simulink model "zcu102_f_200msps_64i_4096c"  (R2021a)
settings: Solver=VariableStepDiscrete  StartTime=0.0  StopTime=10.0  RelTol=1e-3
undefined symbols (not defined in the file or in its folder): datetime, pfb_coeff_gen_calc, posixtime
libraries: xbsIndex_r4 x49646, casper_library_misc x229, casper_library_delays x133, xps_library x20, casper_library_sources x4, xbsReplacements_r4 x4
(part 14 of 502)

== system zcu102_f_200msps_64i_4096c/delay/bus_expand4 ==
  bus_in : Inport
  slice1 : xbsIndex_r4/Slice  nbits=1  boolean_output=on  mode=Upper Bit Location + Width  bit1=-15  base1=MSB of Input  bit0=0  base0=LSB of Input  dbl_ovrd=off  block_type=slice
  slice10 : xbsIndex_r4/Slice  nbits=1  boolean_output=on  mode=Upper Bit Location + Width  bit1=-6  base1=MSB of Input  bit0=0  base0=LSB of Input  dbl_ovrd=off  block_type=slice
  slice11 : xbsIndex_r4/Slice  nbits=1  boolean_output=on  mode=Upper Bit Location + Width  bit1=-5  base1=MSB of Input  bit0=0  base0=LSB of Input  dbl_ovrd=off  block_type=slice
  slice12 : xbsIndex_r4/Slice  nbits=1  boolean_output=on  mode=Upper Bit Location + Width  bit1=-4  base1=MSB of Input  bit0=0  base0=LSB of Input  dbl_ovrd=off  block_type=slice
  slice13 : xbsIndex_r4/Slice  nbits=1  boolean_output=on  mode=Upper Bit Location + Width  bit1=-3  base1=MSB of Input  bit0=0  base0=LSB of Input  dbl_ovrd=off  block_type=slice
  slice14 : xbsIndex_r4/Slice  nbits=1  boolean_output=on  mode=Upper Bit Location + Width  bit1=-2  base1=MSB of Input  bit0=0  base0=LSB of Input  dbl_ovrd=off  block_type=slice
  slice15 : xbsIndex_r4/Slice  nbits=1  boolean_output=on  mode=Upper Bit Location + Width  bit1=-1  base1=MSB of Input  bit0=0  base0=LSB of Input  dbl_ovrd=off  block_type=slice
  slice16 : xbsIndex_r4/Slice  nbits=1  boolean_output=on  mode=Upper Bit Location + Width  bit1=0  base1=MSB of Input  bit0=0  base0=LSB of Input  dbl_ovrd=off  block_type=slice
  slice2 : xbsIndex_r4/Slice  nbits=1  boolean_output=on  mode=Upper Bit Location + Width  bit1=-14  base1=MSB of Input  bit0=0  base0=LSB of Input  dbl_ovrd=off  block_type=slice
  slice3 : xbsIndex_r4/Slice  nbits=1  boolean_output=on  mode=Upper Bit Location + Width  bit1=-13  base1=MSB of Input  bit0=0  base0=LSB of Input  dbl_ovrd=off  block_type=slice
  slice4 : xbsIndex_r4/Slice  nbits=1  boolean_output=on  mode=Upper Bit Location + Width  bit1=-12  base1=MSB of Input  bit0=0  base0=LSB of Input  dbl_ovrd=off  block_type=slice
  slice5 : xbsIndex_r4/Slice  nbits=1  boolean_output=on  mode=Upper Bit Location + Width  bit1=-11  base1=MSB of Input  bit0=0  base0=LSB of Input  dbl_ovrd=off  block_type=slice
  slice6 : xbsIndex_r4/Slice  nbits=1  boolean_output=on  mode=Upper Bit Location + Width  bit1=-10  base1=MSB of Input  bit0=0  base0=LSB of Input  dbl_ovrd=off  block_type=slice
  slice7 : xbsIndex_r4/Slice  nbits=1  boolean_output=on  mode=Upper Bit Location + Width  bit1=-9  base1=MSB of Input  bit0=0  base0=LSB of Input  dbl_ovrd=off  block_type=slice
  slice8 : xbsIndex_r4/Slice  nbits=1  boolean_output=on  mode=Upper Bit Location + Width  bit1=-8  base1=MSB of Input  bit0=0  base0=LSB of Input  dbl_ovrd=off  block_type=slice
  slice9 : xbsIndex_r4/Slice  nbits=1  boolean_output=on  mode=Upper Bit Location + Width  bit1=-7  base1=MSB of Input  bit0=0  base0=LSB of Input  dbl_ovrd=off  block_type=slice
  msb_out16 : Outport
  out15 : Outport  Port=2
  out14 : Outport  Port=3
  out13 : Outport  Port=4
  out12 : Outport  Port=5
  out11 : Outport  Port=6
  out10 : Outport  Port=7
  out9 : Outport  Port=8
  out8 : Outport  Port=9
  out7 : Outport  Port=10
  out6 : Outport  Port=11
  out5 : Outport  Port=12
  out4 : Outport  Port=13
  out3 : Outport  Port=14
  out2 : Outport  Port=15
  lsb_out1 : Outport  Port=16  VectorParamsAs1DForOutWhenUnconnected=off
  wires:
  bus_in/1 -> slice16/1, slice15/1, slice14/1, slice13/1, slice12/1, slice11/1, slice10/1, slice9/1, slice8/1, slice7/1, slice6/1, slice5/1, slice4/1, slice3/1, slice2/1, slice1/1
  slice16/1 -> msb_out16/1
  slice15/1 -> out15/1
  slice14/1 -> out14/1
  slice13/1 -> out13/1
  slice12/1 -> out12/1
  slice11/1 -> out11/1
  slice10/1 -> out10/1
  slice9/1 -> out9/1
  slice8/1 -> out8/1
  slice7/1 -> out7/1
  slice6/1 -> out6/1
  slice5/1 -> out5/1
  slice4/1 -> out4/1
  slice3/1 -> out3/1
  slice2/1 -> out2/1
  slice1/1 -> lsb_out1/1

== system zcu102_f_200msps_64i_4096c/delay/delay0 ==
  sim_1 : Inport
  convert1_1 : DataTypeConversion  OutDataTypeStr=fixdt('ufix13_En0')  LockScale=on
  convert2_1 : DataTypeConversion  OutDataTypeStr=fixdt('ufix13_En0')  ConvertRealWorld=Stored Integer (SI)  LockScale=on
  gain_1 : Gain  OutDataTypeStr=fixdt('ufix32_En0')
  io_delay : xbsIndex_r4/Delay  rst=off  infoeditControl=Selection of Reset will increase slice count due to use of real FFs and instead of SRLs  en=off  latency=0  reg_retiming=on  dbl_ovrd=off  block_type=delay
  reint1 : xbsIndex_r4/Reinterpret  force_arith_type=on  arith_type=Unsigned  force_bin_pt=on  bin_pt=0  block_type=reinterpret
  slice_reg : xbsIndex_r4/Slice  nbits=13  boolean_output=off  mode=Lower Bit Location + Width  bit1=0  base1=MSB of Input  bit0=0  base0=LSB of Input  dbl_ovrd=off  block_type=slice
  zcu102_f_200msps_64i_4096c_delay_delay0_user_data_out : xbsIndex_r4/Gateway In  gui_display_data_type=Fixed-point  arith_type=Unsigned  n_bits=32  bin_pt=0  preci_type=Single  exp_width=8  frac_width=24  rows=1  columns=1  quantization="Round  (unbiased: +/- Inf)"  overflow=Saturate  period=1  UseAsADC=off  ADCChannel='1'  interface=None  automatic_assignment=on  address_offset=hex2dec('00')  intf_name=''  intf_description=''  default_value=0  timing_constraint=None  locs_specified=off  LOCs={}  io_standard={}  inherit_from_input=off  hdl_port=on  dbl_ovrd=off  block_type=gatewayin
  in_reg : Outport  VectorParamsAs1DForOutWhenUnconnected=off
  wires:
  zcu102_f_200msps_64i_4096c_delay_delay0_user_data_out/1 -> io_delay/1
  sim_1/1 -> convert1_1/1
  convert1_1/1 -> convert2_1/1
  convert2_1/1 -> gain_1/1
  gain_1/1 -> zcu102_f_200msps_64i_4096c_delay_delay0_user_data_out/1
  io_delay/1 -> slice_reg/1
  slice_reg/1 -> reint1/1
  reint1/1 -> in_reg/1

== system zcu102_f_200msps_64i_4096c/delay/delay1 ==
  sim_1 : Inport
  convert1_1 : DataTypeConversion  OutDataTypeStr=fixdt('ufix13_En0')  LockScale=on
  convert2_1 : DataTypeConversion  OutDataTypeStr=fixdt('ufix13_En0')  ConvertRealWorld=Stored Integer (SI)  LockScale=on
  gain_1 : Gain  OutDataTypeStr=fixdt('ufix32_En0')
  io_delay : xbsIndex_r4/Delay  rst=off  infoeditControl=Selection of Reset will increase slice count due to use of real FFs and instead of SRLs  en=off  latency=0  reg_retiming=on  dbl_ovrd=off  block_type=delay
  reint1 : xbsIndex_r4/Reinterpret  force_arith_type=on  arith_type=Unsigned  force_bin_pt=on  bin_pt=0  block_type=reinterpret
  slice_reg : xbsIndex_r4/Slice  nbits=13  boolean_output=off  mode=Lower Bit Location + Width  bit1=0  base1=MSB of Input  bit0=0  base0=LSB of Input  dbl_ovrd=off  block_type=slice
  zcu102_f_200msps_64i_4096c_delay_delay1_user_data_out : xbsIndex_r4/Gateway In  gui_display_data_type=Fixed-point  arith_type=Unsigned  n_bits=32  bin_pt=0  preci_type=Single  exp_width=8  frac_width=24  rows=1  columns=1  quantization="Round  (unbiased: +/- Inf)"  overflow=Saturate  period=1  UseAsADC=off  ADCChannel='1'  interface=None  automatic_assignment=on  address_offset=hex2dec('00')  intf_name=''  intf_description=''  default_value=0  timing_constraint=None  locs_specified=off  LOCs={}  io_standard={}  inherit_from_input=off  hdl_port=on  dbl_ovrd=off  block_type=gatewayin
  in_reg : Outport  VectorParamsAs1DForOutWhenUnconnected=off
  wires:
  zcu102_f_200msps_64i_4096c_delay_delay1_user_data_out/1 -> io_delay/1
  sim_1/1 -> convert1_1/1
  convert1_1/1 -> convert2_1/1
  convert2_1/1 -> gain_1/1
  gain_1/1 -> zcu102_f_200msps_64i_4096c_delay_delay1_user_data_out/1
  io_delay/1 -> slice_reg/1
  slice_reg/1 -> reint1/1
  reint1/1 -> in_reg/1

== system zcu102_f_200msps_64i_4096c/delay/delay_en0 ==
  sim_1 : Inport
  convert1_1 : DataTypeConversion  OutDataTypeStr=fixdt('ufix32_En0')  LockScale=on
  convert2_1 : DataTypeConversion  OutDataTypeStr=fixdt('ufix32_En0')  ConvertRealWorld=Stored Integer (SI)  LockScale=on
  gain_1 : Gain  OutDataTypeStr=fixdt('ufix32_En0')
  io_delay : xbsIndex_r4/Delay  rst=off  infoeditControl=Selection of Reset will increase slice count due to use of real FFs and instead of SRLs  en=off  latency=0  reg_retiming=on  dbl_ovrd=off  block_type=delay
  reint1 : xbsIndex_r4/Reinterpret  force_arith_type=on  arith_type=Unsigned  force_bin_pt=on  bin_pt=0  block_type=reinterpret
  slice_reg : xbsIndex_r4/Slice  nbits=32  boolean_output=off  mode=Lower Bit Location + Width  bit1=0  base1=MSB of Input  bit0=0  base0=LSB of Input  dbl_ovrd=off  block_type=slice
  zcu102_f_200msps_64i_4096c_delay_delay_en0_user_data_out : xbsIndex_r4/Gateway In  gui_display_data_type=Fixed-point  arith_type=Unsigned  n_bits=32  bin_pt=0  preci_type=Single  exp_width=8  frac_width=24  rows=1  columns=1  quantization="Round  (unbiased: +/- Inf)"  overflow=Saturate  period=1  UseAsADC=off  ADCChannel='1'  interface=None  automatic_assignment=on  address_offset=hex2dec('00')  intf_name=''  intf_description=''  default_value=0  timing_constraint=None  locs_specified=off  LOCs={}  io_standard={}  inherit_from_input=off  hdl_port=on  dbl_ovrd=off  block_type=gatewayin
  in_reg : Outport  VectorParamsAs1DForOutWhenUnconnected=off
  wires:
  zcu102_f_200msps_64i_4096c_delay_delay_en0_user_data_out/1 -> io_delay/1
  sim_1/1 -> convert1_1/1
  convert1_1/1 -> convert2_1/1
  convert2_1/1 -> gain_1/1
  gain_1/1 -> zcu102_f_200msps_64i_4096c_delay_delay_en0_user_data_out/1
  io_delay/1 -> slice_reg/1
  slice_reg/1 -> reint1/1
  reint1/1 -> in_reg/1

== system zcu102_f_200msps_64i_4096c/delay/delay_en1 ==
  sim_1 : Inport
  convert1_1 : DataTypeConversion  OutDataTypeStr=fixdt('ufix32_En0')  LockScale=on
  convert2_1 : DataTypeConversion  OutDataTypeStr=fixdt('ufix32_En0')  ConvertRealWorld=Stored Integer (SI)  LockScale=on
  gain_1 : Gain  OutDataTypeStr=fixdt('ufix32_En0')
  io_delay : xbsIndex_r4/Delay  rst=off  infoeditControl=Selection of Reset will increase slice count due to use of real FFs and instead of SRLs  en=off  latency=0  reg_retiming=on  dbl_ovrd=off  block_type=delay
  reint1 : xbsIndex_r4/Reinterpret  force_arith_type=on  arith_type=Unsigned  force_bin_pt=on  bin_pt=0  block_type=reinterpret
  slice_reg : xbsIndex_r4/Slice  nbits=32  boolean_output=off  mode=Lower Bit Location + Width  bit1=0  base1=MSB of Input  bit0=0  base0=LSB of Input  dbl_ovrd=off  block_type=slice
  zcu102_f_200msps_64i_4096c_delay_delay_en1_user_data_out : xbsIndex_r4/Gateway In  gui_display_data_type=Fixed-point  arith_type=Unsigned  n_bits=32  bin_pt=0  preci_type=Single  exp_width=8  frac_width=24  rows=1  columns=1  quantization="Round  (unbiased: +/- Inf)"  overflow=Saturate  period=1  UseAsADC=off  ADCChannel='1'  interface=None  automatic_assignment=on  address_offset=hex2dec('00')  intf_name=''  intf_description=''  default_value=0  timing_constraint=None  locs_specified=off  LOCs={}  io_standard={}  inherit_from_input=off  hdl_port=on  dbl_ovrd=off  block_type=gatewayin
  in_reg : Outport  VectorParamsAs1DForOutWhenUnconnected=off
  wires:
  zcu102_f_200msps_64i_4096c_delay_delay_en1_user_data_out/1 -> io_delay/1
  sim_1/1 -> convert1_1/1
  convert1_1/1 -> convert2_1/1
  convert2_1/1 -> gain_1/1
  gain_1/1 -> zcu102_f_200msps_64i_4096c_delay_delay_en1_user_data_out/1
  io_delay/1 -> slice_reg/1
  slice_reg/1 -> reint1/1
  reint1/1 -> in_reg/1

== system zcu102_f_200msps_64i_4096c/delay/delay_store0 ==
  delay_in : Inport
  en0 : Inport  Port=2
  en1 : Inport  Port=3
  en2 : Inport  Port=4
  en3 : Inport  Port=5
  en4 : Inport  Port=6
  en5 : Inport  Port=7
  en6 : Inport  Port=8
  en7 : Inport  Port=9
  en8 : Inport  Port=10
  en9 : Inport  Port=11
  en10 : Inport  Port=12
  en11 : Inport  Port=13
  en12 : Inport  Port=14
  en13 : Inport  Port=15
  en14 : Inport  Port=16
  en15 : Inport  Port=17
  en16 : Inport  Port=18
  en17 : Inport  Port=19
  en18 : Inport  Port=20
  en19 : Inport  Port=21
  en20 : Inport  Port=22
  en21 : Inport  Port=23
  en22 : Inport  Port=24
  en23 : Inport  Port=25
  en24 : Inport  Port=26
  en25 : Inport  Port=27
  en26 : Inport  Port=28
  en27 : Inport  Port=29
  en28 : Inport  Port=30
  en29 : Inport  Port=31
  en30 : Inport  Port=32
  en31 : Inport  Port=33
  Mux{,1..3} (4 alike) : xbsIndex_r4/Mux  inputs=8  en=off  latency=1  precision=Full  arith_type=Unsigned  n_bits=16  bin_pt=14  quantization=Truncate  overflow=Wrap  dbl_ovrd=off  block_type=mux
  Mux4 : xbsIndex_r4/Mux  inputs=4  en=off  latency=1  precision=Full  arith_type=Unsigned  n_bits=16  bin_pt=14  quantization=Truncate  overflow=Wrap  dbl_ovrd=off  block_type=mux
  Register{,1..31} (32 alike) : xbsIndex_r4/Register  init=0  rst=off  en=on  dbl_ovrd=off  block_type=register
  Slice : xbsIndex_r4/Slice  nbits=3  boolean_output=off  mode=Lower Bit Location + Width  bit1=0  base1=MSB of Input  bit0=0  base0=LSB of Input  dbl_ovrd=off  block_type=slice
  Slice1 : xbsIndex_r4/Slice  nbits=2  boolean_output=off  mode=Lower Bit Location + Width  bit1=3  base1=MSB of Input  bit0=3  base0=LSB of Input  dbl_ovrd=off  block_type=slice
  readout : Subsystem  Tag=xps:sw_reg  AncestorBlock=xps_library/Memory/software_register  mask(swreg){io_dir=To Processor, io_delay=0, init_val=0, sample_period=1, names=reg, bitwidths=13, bin_pts=0, arith_types=0, sim_port=on, show_format=on, desc_str=""}
      mask prompts: io_dir "I/O direction"; io_delay "I/O delay"; init_val "Initial Value"; sample_period "Sample period"; names "Bitfield names [msb...lsb]"; bitwidths "Bitfield widths"; bin_pts "Bitfield binary pts"; arith_types "Bitfield types, ufix=0, fix=1, bool=2"; sim_port "Provide sim input/output?"; show_format "Print format string?"; desc_str "Description"
      mask help: A 32-bit software-accessible register. Can be divided into bitfields of varying widths and types using the fields in the block mask.
      mask init:
      | swreg_init(gcb)
      settings: AttributesFormatString=1output:uf13.0 = 13 bits
      mask TypeOptions io_dir: To Processor | From Processor
      mask Callback io_dir:
      | mask_enables = get_param(gcb, 'MaskEnables');
      | mask_names = get_param(gcb, 'MaskNames');
      | if strcmp(get_param(gcb, 'io_dir'), 'To Processor'),
      |     mask_enables{ismember(mask_names, 'sample_period')} = 'off';
      | else
      |     mask_enables{ismember(mask_names, 'sample_period')} = 'on';
      | end
      | set_param(gcb, 'MaskEnables', mask_enables);
      mask DialogControl ParameterTabVar0 Prompt: Setup
  sel : Subsystem  Tag=xps:sw_reg  AncestorBlock=xps_library/Memory/software_register  mask(swreg){io_dir=From Processor, io_delay=0, init_val=0, sample_period=1, names=reg, bitwidths=5, bin_pts=0, arith_types=0, sim_port=on, show_format=on, desc_str=""}
      mask prompts: io_dir "I/O direction"; io_delay "I/O delay"; init_val "Initial Value"; sample_period "Sample period"; names "Bitfield names [msb...lsb]"; bitwidths "Bitfield widths"; bin_pts "Bitfield binary pts"; arith_types "Bitfield types, ufix=0, fix=1, bool=2"; sim_port "Provide sim input/output?"; show_format "Print format string?"; desc_str "Description"
      mask help: A 32-bit software-accessible register. Can be divided into bitfields of varying widths and types using the fields in the block mask.
      mask init:
      | swreg_init(gcb)
      settings: AttributesFormatString=1input:uf5.0 = 5 bits
      mask TypeOptions io_dir: To Processor | From Processor
      mask Callback io_dir:
      | mask_enables = get_param(gcb, 'MaskEnables');
      | mask_names = get_param(gcb, 'MaskNames');
      | if strcmp(get_param(gcb, 'io_dir'), 'To Processor'),
      |     mask_enables{ismember(mask_names, 'sample_period')} = 'off';
      | else
      |     mask_enables{ismember(mask_names, 'sample_period')} = 'on';
      | end
      | set_param(gcb, 'MaskEnables', mask_enables);
      mask DialogControl ParameterTabVar0 Prompt: Setup
  delay0 : Outport  VectorParamsAs1DForOutWhenUnconnected=off
  delay1 : Outport  Port=2  VectorParamsAs1DForOutWhenUnconnected=off
  delay2 : Outport  Port=3  VectorParamsAs1DForOutWhenUnconnected=off
  delay3 : Outport  Port=4  VectorParamsAs1DForOutWhenUnconnected=off
  delay4 : Outport  Port=5  VectorParamsAs1DForOutWhenUnconnected=off
  delay5 : Outport  Port=6  VectorParamsAs1DForOutWhenUnconnected=off
  delay6 : Outport  Port=7  VectorParamsAs1DForOutWhenUnconnected=off
  delay7 : Outport  Port=8  VectorParamsAs1DForOutWhenUnconnected=off
  delay8 : Outport  Port=9  VectorParamsAs1DForOutWhenUnconnected=off
  delay9 : Outport  Port=10  VectorParamsAs1DForOutWhenUnconnected=off
  delay10 : Outport  Port=11  VectorParamsAs1DForOutWhenUnconnected=off
  delay11 : Outport  Port=12  VectorParamsAs1DForOutWhenUnconnected=off
  delay12 : Outport  Port=13  VectorParamsAs1DForOutWhenUnconnected=off
  delay13 : Outport  Port=14  VectorParamsAs1DForOutWhenUnconnected=off
  delay14 : Outport  Port=15  VectorParamsAs1DForOutWhenUnconnected=off
  delay15 : Outport  Port=16  VectorParamsAs1DForOutWhenUnconnected=off
  delay16 : Outport  Port=17  VectorParamsAs1DForOutWhenUnconnected=off
  delay17 : Outport  Port=18  VectorParamsAs1DForOutWhenUnconnected=off
  delay18 : Outport  Port=19  VectorParamsAs1DForOutWhenUnconnected=off
  delay19 : Outport  Port=20  VectorParamsAs1DForOutWhenUnconnected=off
  delay20 : Outport  Port=21  VectorParamsAs1DForOutWhenUnconnected=off
  delay21 : Outport  Port=22  VectorParamsAs1DForOutWhenUnconnected=off
  delay22 : Outport  Port=23  VectorParamsAs1DForOutWhenUnconnected=off
  delay23 : Outport  Port=24  VectorParamsAs1DForOutWhenUnconnected=off
  delay24 : Outport  Port=25  VectorParamsAs1DForOutWhenUnconnected=off
  delay25 : Outport  Port=26  VectorParamsAs1DForOutWhenUnconnected=off
  delay26 : Outport  Port=27  VectorParamsAs1DForOutWhenUnconnected=off
  delay27 : Outport  Port=28  VectorParamsAs1DForOutWhenUnconnected=off
  delay28 : Outport  Port=29  VectorParamsAs1DForOutWhenUnconnected=off
  delay29 : Outport  Port=30  VectorParamsAs1DForOutWhenUnconnected=off
  delay30 : Outport  Port=31  VectorParamsAs1DForOutWhenUnconnected=off
  delay31 : Outport  Port=32  VectorParamsAs1DForOutWhenUnconnected=off
  wires:
  Register/1 -> Mux/2, delay0/1
  en0/1 -> Register/2
  delay_in/1 -> [delay]
  [delay] -> Register/1
  [delay] -> Register1/1
  en1/1 -> Register1/2
  Register1/1 -> Mux/3, delay1/1
  Register2/1 -> Mux/4, delay2/1
  en2/1 -> Register2/2
  [delay] -> Register2/1
  [delay] -> Register3/1
  en3/1 -> Register3/2
  Register3/1 -> Mux/5, delay3/1
  Register4/1 -> Mux/6, delay4/1
  en4/1 -> Register4/2
  [delay] -> Register4/1
  [delay] -> Register5/1
  en5/1 -> Register5/2
  Register5/1 -> Mux/7, delay5/1
  Register6/1 -> Mux/8, delay6/1
  en6/1 -> Register6/2
  [delay] -> Register6/1
  [delay] -> Register7/1
  en7/1 -> Register7/2
  Register7/1 -> Mux/9, delay7/1
  en15/1 -> Register15/2
  [delay] -> Register15/1
  [delay] -> Register14/1
  en14/1 -> Register14/2
  en13/1 -> Register13/2
  [delay] -> Register13/1
  [delay] -> Register12/1
  en12/1 -> Register12/2
  en11/1 -> Register11/2
  [delay] -> Register11/1
  [delay] -> Register10/1
  en10/1 -> Register10/2
  en9/1 -> Register9/2
  [delay] -> Register9/1
  [delay] -> Register8/1
  en8/1 -> Register8/2
  en16/1 -> Register22/2
  [delay] -> Register22/1
  [delay] -> Register23/1
  en17/1 -> Register23/2
  en18/1 -> Register16/2
  [delay] -> Register16/1
  [delay] -> Register17/1
  en19/1 -> Register17/2
  en20/1 -> Register18/2
  [delay] -> Register18/1
  [delay] -> Register19/1
  en21/1 -> Register19/2
  en22/1 -> Register20/2
  [delay] -> Register20/1
  [delay] -> Register21/1
  en23/1 -> Register21/2
  en31/1 -> Register29/2
  [delay] -> Register29/1
  [delay] -> Register28/1
  en30/1 -> Register28/2
  en29/1 -> Register27/2
  [delay] -> Register27/1
  [delay] -> Register26/1
  en28/1 -> Register26/2
  en27/1 -> Register25/2
  [delay] -> Register25/1
  [delay] -> Register24/1
  en26/1 -> Register24/2
  en25/1 -> Register31/2
  [delay] -> Register31/1
  [delay] -> Register30/1
  en24/1 -> Register30/2
  Register15/1 -> Mux1/9, delay15/1
  Register14/1 -> Mux1/8, delay14/1
  Register13/1 -> Mux1/7, delay13/1
  Register12/1 -> Mux1/6, delay12/1
  Register11/1 -> Mux1/5, delay11/1
  Register10/1 -> Mux1/4, delay10/1
  Register9/1 -> Mux1/3, delay9/1
  Register8/1 -> Mux1/2, delay8/1
  Register22/1 -> Mux2/2, delay16/1
  Register23/1 -> delay17/1, Mux2/3
  Register16/1 -> delay18/1, Mux2/4
  Register17/1 -> Mux2/5, delay19/1
  Register18/1 -> Mux2/6, delay20/1
  Register19/1 -> Mux2/7, delay21/1
  Register20/1 -> Mux2/8, delay22/1
  Register21/1 -> Mux2/9, delay23/1
  Register29/1 -> Mux3/9, delay31/1
  Register28/1 -> Mux3/8, delay30/1
  Register27/1 -> Mux3/7, delay29/1
  Register26/1 -> Mux3/6, delay28/1
  Register25/1 -> Mux3/5, delay27/1
  Register24/1 -> Mux3/4, delay26/1
  Register31/1 -> Mux3/3, delay25/1
  Register30/1 -> Mux3/2, delay24/1
  Mux/1 -> Mux4/2
  Mux1/1 -> Mux4/3
  Mux2/1 -> Mux4/4
  Mux3/1 -> Mux4/5
  Mux4/1 -> readout/1(out_reg)
  sel/1(in_reg) -> Slice1/1, Slice/1
  Slice1/1 -> [sel_msb]
  Slice/1 -> [sel_lsb]
  [sel_lsb] -> Mux/1
  [sel_lsb] -> Mux1/1
  [sel_lsb] -> Mux2/1
  [sel_lsb] -> Mux3/1
  [sel_msb] -> Mux4/1

== system zcu102_f_200msps_64i_4096c/delay/delay_store1 ==
  delay_in : Inport
  en0 : Inport  Port=2
  en1 : Inport  Port=3
  en2 : Inport  Port=4
  en3 : Inport  Port=5
  en4 : Inport  Port=6
  en5 : Inport  Port=7
  en6 : Inport  Port=8
  en7 : Inport  Port=9
  en8 : Inport  Port=10
  en9 : Inport  Port=11
  en10 : Inport  Port=12
  en11 : Inport  Port=13
  en12 : Inport  Port=14
  en13 : Inport  Port=15
  en14 : Inport  Port=16
  en15 : Inport  Port=17
  en16 : Inport  Port=18
  en17 : Inport  Port=19
  en18 : Inport  Port=20
  en19 : Inport  Port=21
  en20 : Inport  Port=22
  en21 : Inport  Port=23
  en22 : Inport  Port=24
  en23 : Inport  Port=25
  en24 : Inport  Port=26
  en25 : Inport  Port=27
  en26 : Inport  Port=28
  en27 : Inport  Port=29
  en28 : Inport  Port=30
  en29 : Inport  Port=31
  en30 : Inport  Port=32
  en31 : Inport  Port=33
  Mux{,1..3} (4 alike) : xbsIndex_r4/Mux  inputs=8  en=off  latency=1  precision=Full  arith_type=Unsigned  n_bits=16  bin_pt=14  quantization=Truncate  overflow=Wrap  dbl_ovrd=off  block_type=mux
  Mux4 : xbsIndex_r4/Mux  inputs=4  en=off  latency=1  precision=Full  arith_type=Unsigned  n_bits=16  bin_pt=14  quantization=Truncate  overflow=Wrap  dbl_ovrd=off  block_type=mux
  Register{,1..31} (32 alike) : xbsIndex_r4/Register  init=0  rst=off  en=on  dbl_ovrd=off  block_type=register
  Slice : xbsIndex_r4/Slice  nbits=3  boolean_output=off  mode=Lower Bit Location + Width  bit1=0  base1=MSB of Input  bit0=0  base0=LSB of Input  dbl_ovrd=off  block_type=slice
  Slice1 : xbsIndex_r4/Slice  nbits=2  boolean_output=off  mode=Lower Bit Location + Width  bit1=3  base1=MSB of Input  bit0=3  base0=LSB of Input  dbl_ovrd=off  block_type=slice
  readout : Subsystem  Tag=xps:sw_reg  AncestorBlock=xps_library/Memory/software_register  mask(swreg){io_dir=To Processor, io_delay=0, init_val=0, sample_period=1, names=reg, bitwidths=13, bin_pts=0, arith_types=0, sim_port=on, show_format=on, desc_str=""}
      mask prompts: io_dir "I/O direction"; io_delay "I/O delay"; init_val "Initial Value"; sample_period "Sample period"; names "Bitfield names [msb...lsb]"; bitwidths "Bitfield widths"; bin_pts "Bitfield binary pts"; arith_types "Bitfield types, ufix=0, fix=1, bool=2"; sim_port "Provide sim input/output?"; show_format "Print format string?"; desc_str "Description"
      mask help: A 32-bit software-accessible register. Can be divided into bitfields of varying widths and types using the fields in the block mask.
      mask init:
      | swreg_init(gcb)
      settings: AttributesFormatString=1output:uf13.0 = 13 bits
      mask TypeOptions io_dir: To Processor | From Processor
      mask Callback io_dir:
      | mask_enables = get_param(gcb, 'MaskEnables');
      | mask_names = get_param(gcb, 'MaskNames');
      | if strcmp(get_param(gcb, 'io_dir'), 'To Processor'),
      |     mask_enables{ismember(mask_names, 'sample_period')} = 'off';
      | else
      |     mask_enables{ismember(mask_names, 'sample_period')} = 'on';
      | end
      | set_param(gcb, 'MaskEnables', mask_enables);
      mask DialogControl ParameterTabVar0 Prompt: Setup
  sel : Subsystem  Tag=xps:sw_reg  AncestorBlock=xps_library/Memory/software_register  mask(swreg){io_dir=From Processor, io_delay=0, init_val=0, sample_period=1, names=reg, bitwidths=5, bin_pts=0, arith_types=0, sim_port=on, show_format=on, desc_str=""}
      mask prompts: io_dir "I/O direction"; io_delay "I/O delay"; init_val "Initial Value"; sample_period "Sample period"; names "Bitfield names [msb...lsb]"; bitwidths "Bitfield widths"; bin_pts "Bitfield binary pts"; arith_types "Bitfield types, ufix=0, fix=1, bool=2"; sim_port "Provide sim input/output?"; show_format "Print format string?"; desc_str "Description"
      mask help: A 32-bit software-accessible register. Can be divided into bitfields of varying widths and types using the fields in the block mask.
      mask init:
      | swreg_init(gcb)
      settings: AttributesFormatString=1input:uf5.0 = 5 bits
      mask TypeOptions io_dir: To Processor | From Processor
      mask Callback io_dir:
      | mask_enables = get_param(gcb, 'MaskEnables');
      | mask_names = get_param(gcb, 'MaskNames');
      | if strcmp(get_param(gcb, 'io_dir'), 'To Processor'),
      |     mask_enables{ismember(mask_names, 'sample_period')} = 'off';
      | else
      |     mask_enables{ismember(mask_names, 'sample_period')} = 'on';
      | end
      | set_param(gcb, 'MaskEnables', mask_enables);
      mask DialogControl ParameterTabVar0 Prompt: Setup
  delay0 : Outport  VectorParamsAs1DForOutWhenUnconnected=off
  delay1 : Outport  Port=2  VectorParamsAs1DForOutWhenUnconnected=off
  delay2 : Outport  Port=3  VectorParamsAs1DForOutWhenUnconnected=off
  delay3 : Outport  Port=4  VectorParamsAs1DForOutWhenUnconnected=off
  delay4 : Outport  Port=5  VectorParamsAs1DForOutWhenUnconnected=off
  delay5 : Outport  Port=6  VectorParamsAs1DForOutWhenUnconnected=off
  delay6 : Outport  Port=7  VectorParamsAs1DForOutWhenUnconnected=off
  delay7 : Outport  Port=8  VectorParamsAs1DForOutWhenUnconnected=off
  delay8 : Outport  Port=9  VectorParamsAs1DForOutWhenUnconnected=off
  delay9 : Outport  Port=10  VectorParamsAs1DForOutWhenUnconnected=off
  delay10 : Outport  Port=11  VectorParamsAs1DForOutWhenUnconnected=off
  delay11 : Outport  Port=12  VectorParamsAs1DForOutWhenUnconnected=off
  delay12 : Outport  Port=13  VectorParamsAs1DForOutWhenUnconnected=off
  delay13 : Outport  Port=14  VectorParamsAs1DForOutWhenUnconnected=off
  delay14 : Outport  Port=15  VectorParamsAs1DForOutWhenUnconnected=off
  delay15 : Outport  Port=16  VectorParamsAs1DForOutWhenUnconnected=off
  delay16 : Outport  Port=17  VectorParamsAs1DForOutWhenUnconnected=off
  delay17 : Outport  Port=18  VectorParamsAs1DForOutWhenUnconnected=off
  delay18 : Outport  Port=19  VectorParamsAs1DForOutWhenUnconnected=off
  delay19 : Outport  Port=20  VectorParamsAs1DForOutWhenUnconnected=off
  delay20 : Outport  Port=21  VectorParamsAs1DForOutWhenUnconnected=off
  delay21 : Outport  Port=22  VectorParamsAs1DForOutWhenUnconnected=off
  delay22 : Outport  Port=23  VectorParamsAs1DForOutWhenUnconnected=off
  delay23 : Outport  Port=24  VectorParamsAs1DForOutWhenUnconnected=off
  delay24 : Outport  Port=25  VectorParamsAs1DForOutWhenUnconnected=off
  delay25 : Outport  Port=26  VectorParamsAs1DForOutWhenUnconnected=off
  delay26 : Outport  Port=27  VectorParamsAs1DForOutWhenUnconnected=off
  delay27 : Outport  Port=28  VectorParamsAs1DForOutWhenUnconnected=off
  delay28 : Outport  Port=29  VectorParamsAs1DForOutWhenUnconnected=off
  delay29 : Outport  Port=30  VectorParamsAs1DForOutWhenUnconnected=off
  delay30 : Outport  Port=31  VectorParamsAs1DForOutWhenUnconnected=off
  delay31 : Outport  Port=32  VectorParamsAs1DForOutWhenUnconnected=off
  wires:
  [sel_msb] -> Mux4/1
  [sel_lsb] -> Mux3/1
  [sel_lsb] -> Mux2/1
  [sel_lsb] -> Mux1/1
  [sel_lsb] -> Mux/1
  Slice/1 -> [sel_lsb]
  Slice1/1 -> [sel_msb]
  sel/1(in_reg) -> Slice/1, Slice1/1
  Mux4/1 -> readout/1(out_reg)
  Mux3/1 -> Mux4/5
  Mux2/1 -> Mux4/4
  Mux1/1 -> Mux4/3
  Mux/1 -> Mux4/2
  Register30/1 -> delay24/1, Mux3/2
  Register31/1 -> delay25/1, Mux3/3
  Register24/1 -> delay26/1, Mux3/4
  Register25/1 -> delay27/1, Mux3/5
  Register26/1 -> delay28/1, Mux3/6
  Register27/1 -> delay29/1, Mux3/7
  Register28/1 -> delay30/1, Mux3/8
  Register29/1 -> delay31/1, Mux3/9
  Register21/1 -> delay23/1, Mux2/9
  Register20/1 -> delay22/1, Mux2/8
  Register19/1 -> delay21/1, Mux2/7
  Register18/1 -> delay20/1, Mux2/6
  Register17/1 -> delay19/1, Mux2/5
  Register16/1 -> Mux2/4, delay18/1
  Register23/1 -> Mux2/3, delay17/1
  Register22/1 -> delay16/1, Mux2/2
  Register8/1 -> delay8/1, Mux1/2
  Register9/1 -> delay9/1, Mux1/3
  Register10/1 -> delay10/1, Mux1/4
  Register11/1 -> delay11/1, Mux1/5
  Register12/1 -> delay12/1, Mux1/6
  Register13/1 -> delay13/1, Mux1/7
  Register14/1 -> delay14/1, Mux1/8
  Register15/1 -> delay15/1, Mux1/9
  en24/1 -> Register30/2
  [delay] -> Register30/1
  [delay] -> Register31/1
  en25/1 -> Register31/2
  en26/1 -> Register24/2
  [delay] -> Register24/1
  [delay] -> Register25/1
  en27/1 -> Register25/2
  en28/1 -> Register26/2
  [delay] -> Register26/1
  [delay] -> Register27/1
  en29/1 -> Register27/2
  en30/1 -> Register28/2
  [delay] -> Register28/1
  [delay] -> Register29/1
  en31/1 -> Register29/2
  en23/1 -> Register21/2
  [delay] -> Register21/1
  [delay] -> Register20/1
  en22/1 -> Register20/2
  en21/1 -> Register19/2
  [delay] -> Register19/1
  [delay] -> Register18/1
  en20/1 -> Register18/2
  en19/1 -> Register17/2
  [delay] -> Register17/1
  [delay] -> Register16/1
  en18/1 -> Register16/2
  en17/1 -> Register23/2
  [delay] -> Register23/1
  [delay] -> Register22/1
  en16/1 -> Register22/2
  en8/1 -> Register8/2
  [delay] -> Register8/1
  [delay] -> Register9/1
  en9/1 -> Register9/2
  en10/1 -> Register10/2
  [delay] -> Register10/1
  [delay] -> Register11/1
  en11/1 -> Register11/2
  en12/1 -> Register12/2
  [delay] -> Register12/1
  [delay] -> Register13/1
  en13/1 -> Register13/2
  en14/1 -> Register14/2
  [delay] -> Register14/1
  [delay] -> Register15/1
  en15/1 -> Register15/2
  Register7/1 -> delay7/1, Mux/9
  en7/1 -> Register7/2
  [delay] -> Register7/1
  [delay] -> Register6/1
  en6/1 -> Register6/2
  Register6/1 -> delay6/1, Mux/8
  Register5/1 -> delay5/1, Mux/7
  en5/1 -> Register5/2
  [delay] -> Register5/1
  [delay] -> Register4/1
  en4/1 -> Register4/2
  Register4/1 -> delay4/1, Mux/6
  Register3/1 -> delay3/1, Mux/5
  en3/1 -> Register3/2
  [delay] -> Register3/1
  [delay] -> Register2/1
  en2/1 -> Register2/2
  Register2/1 -> delay2/1, Mux/4
  Register1/1 -> delay1/1, Mux/3
  en1/1 -> Register1/2
  [delay] -> Register1/1
  [delay] -> Register/1
  delay_in/1 -> [delay]
  en0/1 -> Register/2
  Register/1 -> delay0/1, Mux/2

== system zcu102_f_200msps_64i_4096c/delay/delays ==
  addr : Inport
  data_in : Inport  Port=2
  we : Inport  Port=3
  Terminator : Terminator
  Terminator1 : Terminator
  Terminator2 : Terminator
  calc_add : Subsystem  mask{data_width=32, addr_width=6, sample_rate=sample_rate}
      mask prompts: data_width "data width"; addr_width "address width"; sample_rate "sample rate"
  convert_addr : xbsIndex_r4/Convert  gui_display_data_type=Fixed-point  arith_type=Unsigned  n_bits=addr_width  bin_pt=0  float_type=Single  exp_bits=8  fraction_bits=24  quantization=Truncate  overflow=Wrap  en=off  latency=0  pipeline=off  dbl_ovrd=off  block_type=convert
      settings: xl_area=[0, 0, 0, 0, 0, 0, 0]
  convert_din1 : xbsIndex_r4/Convert  gui_display_data_type=Fixed-point  arith_type=Unsigned  n_bits=32  bin_pt=0  float_type=Single  exp_bits=8  fraction_bits=24  quantization=Truncate  overflow=Wrap  en=off  latency=0  pipeline=off  dbl_ovrd=off  block_type=convert
      settings: xl_area=[0, 0, 0, 0, 0, 0, 0]
  convert_we : xbsIndex_r4/Convert  gui_display_data_type=Fixed-point  arith_type=Unsigned  n_bits=1  bin_pt=0  float_type=Single  exp_bits=8  fraction_bits=24  quantization=Truncate  overflow=Wrap  en=off  latency=0  pipeline=off  dbl_ovrd=off  block_type=convert
      settings: xl_area=[0, 0, 0, 0, 0, 0, 0]
  munge_in : Subsystem  AncestorBlock=casper_library_flow_control/munge  mask(munge){divisions=1, div_size=32, order=[0], arith_type_out=Unsigned, bin_pt_out=0}
      mask prompts: divisions "Number of divisions"; div_size "Division size (bits)"; order "Division packing order"; arith_type_out "Output arithmetic type"; bin_pt_out "Output binary point"
      mask help: Splits an input bus into the specified number of divisions and then recombines them in the order specified (most significant division = 0,least = number divisions-1)
      mask init:
      | munge_init(gcb, ...
      |     'divisions', divisions, ...
      |     'div_size', div_size, ...
      |     'order', order, ...
      |     'arith_type_out', arith_type_out, ...
      |     'bin_pt_out', bin_pt_out);
      settings: AttributesFormatString=|
      | split:32
      | join:0
      | Unsigned [32,0]
      mask TypeOptions arith_type_out: Unsigned | Signed  (2's comp) | Floating-point
  munge_out : Subsystem  AncestorBlock=casper_library_flow_control/munge  mask(munge){divisions=1, div_size=32, order=[0], arith_type_out=Unsigned, bin_pt_out=0}
      mask prompts: divisions "Number of divisions"; div_size "Division size (bits)"; order "Division packing order"; arith_type_out "Output arithmetic type"; bin_pt_out "Output binary point"
      mask help: Splits an input bus into the specified number of divisions and then recombines them in the order specified (most significant division = 0,least = number divisions-1)
      mask init:
      | munge_init(gcb, ...
      |     'divisions', divisions, ...
      |     'div_size', div_size, ...
      |     'order', order, ...
      |     'arith_type_out', arith_type_out, ...
      |     'bin_pt_out', bin_pt_out);
      settings: AttributesFormatString=|
      | split:32
      | join:0
      | Unsigned [32,0]
      mask TypeOptions arith_type_out: Unsigned | Signed  (2's comp) | Floating-point
  sim_mem : Subsystem  mask{arith_type=Unsigned, addr_width=6, data_width=32, data_bin_pt=0, init_vals=[0   1   2   3   4   5   6   7   8   9  10  11  12  13  14  15  16  17  18  19  20  21  22  23  24  25  26  27  28  29  30  31  32  33  34  35  36  37  38  39  40  41  42  43  44  45  46  47  48  49  50  51  52  53  54  55  56  57  58  59  60  61  62  63], sample_rate=1, csp_latency=1}
      mask prompts: arith_type "Output Data Type"; addr_width "Address width"; data_width "Data width"; data_bin_pt "Data Binary Point"; init_vals "Initial values"; sample_rate "Sample rate"; csp_latency "Latency"
      mask init:
      | % set_param([gcb, '/data_to_xilinx'], 'arith_type', arith_type)
      | % set_param([gcb, '/data_to_xilinx'], 'n_bits', get_param(gcb, 'data_width'))
      mask TypeOptions arith_type: Unsigned | Signed  (2's comp)
      mask TypeOptions data_width: 8 | 16 | 32 | 64 | 128
  snap2_f_200msps_64i_4096c_delay_delays_addr : xbsIndex_r4/Gateway Out  inherit_from_input=off  hdl_port=on  rows=1  columns=1  UseAsDAC=off  DACChannel='1'  interface=None  automatic_assignment=on  address_offset=hex2dec('00')  intf_name=''  intf_description=''  timing_constraint=None  locs_specified=off  LOCs={'A5','B9','A9','C1','A3','G6','E9','A6','A7','A8','B8','B1','B3','D9','B5','B4','B6','C2'}  io_standard={}  block_type=gatewayout
      settings: infoedit="Gateway out block.  Converts Xilinx fixed point inputs into ouputs of type Simulink integer, double, or fixed point.<P><P>Hardware notes:  In hardware these blocks become top level output ports or are discarded, depending on how they are configured."  sginterface={'Nallatech'=>{'xdspkit'=>{'non_memory_mapped_port'=>'uprev_Shared_BRAM_addr'}},'Xilinx'=>{'jtaghwcosim'=>{'non_memory_mapped_port'=>'uprev_Shared_BRAM_addr'},'xdspkit'=>{'non_memory_mapped_port'=>'uprev_Shared_BRAM_addr'}}}  xl_area=[0, 0, 0, 0, 0, 0, 0]
  snap2_f_200msps_64i_4096c_delay_delays_data_in : xbsIndex_r4/Gateway Out  inherit_from_input=off  hdl_port=on  rows=1  columns=1  UseAsDAC=off  DACChannel='1'  interface=None  automatic_assignment=on  address_offset=hex2dec('00')  intf_name=''  intf_description=''  timing_constraint=None  locs_specified=off  LOCs={'B21','B22','A22','B23','B19','C19','A20','A21','A23','B24','A24','A25','E18','D18','C18','B18'}  io_standard={}  block_type=gatewayout
      settings: infoedit="Gateway out block.  Converts Xilinx fixed point inputs into ouputs of type Simulink integer, double, or fixed point.<P><P>Hardware notes:  In hardware these blocks become top level output ports or are discarded, depending on how they are configured."  sginterface={'Nallatech'=>{'xdspkit'=>{'non_memory_mapped_port'=>'uprev_Shared_BRAM_data_in'}},'Xilinx'=>{'jtaghwcosim'=>{'non_memory_mapped_port'=>'uprev_Shared_BRAM_data_in'},'xdspkit'=>{'non_memory_mapped_port'=>'uprev_Shared_BRAM_data_in'}}}  xl_area=[0, 0, 0, 0, 0, 0, 0]
  snap2_f_200msps_64i_4096c_delay_delays_data_out : xbsIndex_r4/Gateway In  gui_display_data_type=Fixed-point  arith_type=Unsigned  n_bits=32  bin_pt=0  preci_type=Single  exp_width=8  frac_width=24  rows=1  columns=1  quantization=Truncate  overflow=Wrap  period=sample_rate  UseAsADC=off  ADCChannel='1'  interface=None  automatic_assignment=on  address_offset=hex2dec('00')  intf_name=''  intf_description=''  default_value=0  timing_constraint=None  locs_specified=off  LOCs={}  io_standard={}  inherit_from_input=off  hdl_port=on  dbl_ovrd=off  block_type=gatewayin
      settings: xl_area=[0, 0, 0, 0, 0, 0, 0]
  snap2_f_200msps_64i_4096c_delay_delays_we : xbsIndex_r4/Gateway Out  inherit_from_input=off  hdl_port=on  rows=1  columns=1  UseAsDAC=off  DACChannel='1'  interface=None  automatic_assignment=on  address_offset=hex2dec('00')  intf_name=''  intf_description=''  timing_constraint=None  locs_specified=off  LOCs={'A14'}  io_standard={}  block_type=gatewayout
      settings: infoedit="Gateway out block.  Converts Xilinx fixed point inputs into ouputs of type Simulink integer, double, or fixed point.<P><P>Hardware notes:  In hardware these blocks become top level output ports or are discarded, depending on how they are configured."  xl_area=[0, 0, 0, 0, 0, 0, 0]
  data_out : Outport  VectorParamsAs1DForOutWhenUnconnected=off
  wires:
  munge_in/1(dout) -> convert_din1/1, sim_mem/2(data)
  we/1 -> convert_we/1, sim_mem/3(we)
  addr/1 -> calc_add/1(in)
  convert_we/1 -> snap2_f_200msps_64i_4096c_delay_delays_we/1
  snap2_f_200msps_64i_4096c_delay_delays_addr/1 -> Terminator/1
  snap2_f_200msps_64i_4096c_delay_delays_data_in/1 -> Terminator1/1
  snap2_f_200msps_64i_4096c_delay_delays_we/1 -> Terminator2/1
  calc_add/1(out) -> convert_addr/1, sim_mem/1(addr)
  data_in/1 -> munge_in/1(din)
  convert_din1/1 -> snap2_f_200msps_64i_4096c_delay_delays_data_in/1
  convert_addr/1 -> snap2_f_200msps_64i_4096c_delay_delays_addr/1
  snap2_f_200msps_64i_4096c_delay_delays_data_out/1 -> munge_out/1(din)
  sim_mem/1(data_out) -> snap2_f_200msps_64i_4096c_delay_delays_data_out/1
  munge_out/1(dout) -> data_out/1

== system zcu102_f_200msps_64i_4096c/delay/max_delay ==
  out_reg : Inport
  assert_reg : xbsIndex_r4/Assert  assert_type=on  type_source=Explicitly  gui_display_data_type=Fixed-point  arith_type=Unsigned  n_bits=32  bin_pt=0  preci_type=Single  exp_width=8  frac_width=24  assert_rate=off  rate_source=Explicitly  period=1  output_port=on  block_type=assert
  cast_gw : xbsIndex_r4/Convert  gui_display_data_type=Fixed-point  arith_type=Unsigned  n_bits=32  bin_pt=0  float_type=Single  exp_bits=8  fraction_bits=24  quantization=Truncate  overflow=Wrap  en=off  latency=1  pipeline=off  dbl_ovrd=off  block_type=convert
  io_delay : xbsIndex_r4/Delay  rst=off  infoeditControl=Selection of Reset will increase slice count due to use of real FFs and instead of SRLs  en=off  latency=0  reg_retiming=on  dbl_ovrd=off  block_type=delay
  reint1 : xbsIndex_r4/Reinterpret  force_arith_type=on  arith_type=Unsigned  force_bin_pt=on  bin_pt=0  block_type=reinterpret
  sim_out : Terminator
  sim_out_reg_gw : xbsIndex_r4/Gateway Out  inherit_from_input=off  hdl_port=off  rows=1  columns=1  UseAsDAC=off  DACChannel='1'  interface=None  automatic_assignment=on  address_offset=hex2dec('00')  intf_name=''  intf_description=''  timing_constraint=None  locs_specified=off  LOCs={}  io_standard={}  block_type=gatewayout
  sim_out_reg_sim_delay : xbsIndex_r4/Delay  rst=off  infoeditControl=Selection of Reset will increase slice count due to use of real FFs and instead of SRLs  en=off  latency=1  reg_retiming=on  dbl_ovrd=off  block_type=delay
  zcu102_f_200msps_64i_4096c_delay_max_delay_user_data_in : xbsIndex_r4/Gateway Out  inherit_from_input=off  hdl_port=on  rows=1  columns=1  UseAsDAC=off  DACChannel='1'  interface=None  automatic_assignment=on  address_offset=hex2dec('00')  intf_name=''  intf_description=''  timing_constraint=None  locs_specified=off  LOCs={}  io_standard={}  block_type=gatewayout
  sim_out_reg : Outport  VectorParamsAs1DForOutWhenUnconnected=off
  wires:
  sim_out_reg_sim_delay/1 -> sim_out_reg_gw/1
  sim_out_reg_gw/1 -> sim_out_reg/1
  io_delay/1 -> cast_gw/1
  cast_gw/1 -> zcu102_f_200msps_64i_4096c_delay_max_delay_user_data_in/1
  zcu102_f_200msps_64i_4096c_delay_max_delay_user_data_in/1 -> sim_out/1
  out_reg/1 -> sim_out_reg_sim_delay/1, assert_reg/1
  assert_reg/1 -> reint1/1
  reint1/1 -> io_delay/1

== system zcu102_f_200msps_64i_4096c/eq/bus_create2 ==
  in1 : Inport
  in2 : Inport  Port=2
  in3 : Inport  Port=3
  in4 : Inport  Port=4
  concatenate : xbsIndex_r4/Concat  num_inputs=4  dbl_ovrd=off  block_type=concat
  reinterpret{1..4} (4 alike) : xbsIndex_r4/Reinterpret  force_arith_type=on  arith_type=Unsigned  force_bin_pt=on  bin_pt=0  block_type=reinterpret
      reinterpret1 settings: infoedit="Changes signal type without altering the binary representation.   You can changed the signal between signed and unsigned, and relocate the binary point.<br><br>Hardware notes: In hardware this block costs nothing.<br><br>Example:  Suppose the input is 6 bits wide, signed, with 2 fractional bits, and the output is forced to unsigned with 0 fractional bits.  Then an input of -2.0 (1110.00 in binary 2's complement) becomes an output of 56 (111000 in binary)."
      reinterpret2 settings: infoedit="Changes signal type without altering the binary representation.   You can changed the signal between signed and unsigned, and relocate the binary point.<br><br>Hardware notes: In hardware this block costs nothing.<br><br>Example:  Suppose the input is 6 bits wide, signed, with 2 fractional bits, and the output is forced to unsigned with 0 fractional bits.  Then an input of -2.0 (1110.00 in binary 2's complement) becomes an output of 56 (111000 in binary)."
  bus_out : Outport  VectorParamsAs1DForOutWhenUnconnected=off
  wires:
  concatenate/1 -> bus_out/1
  in1/1 -> reinterpret1/1
  reinterpret1/1 -> concatenate/1
  in2/1 -> reinterpret2/1
  reinterpret2/1 -> concatenate/2
  in3/1 -> reinterpret3/1
  reinterpret3/1 -> concatenate/3
  in4/1 -> reinterpret4/1
  reinterpret4/1 -> concatenate/4

== system zcu102_f_200msps_64i_4096c/eq/bus_expand4 ==
  bus_in : Inport
  reinterpret{1..4} (4 alike) : xbsIndex_r4/Reinterpret  force_arith_type=on  arith_type=Unsigned  force_bin_pt=on  bin_pt=0  block_type=reinterpret
  slice1 : xbsIndex_r4/Slice  nbits=288  boolean_output=off  mode=Upper Bit Location + Width  bit1=-864  base1=MSB of Input  bit0=0  base0=LSB of Input  dbl_ovrd=off  block_type=slice
  slice2 : xbsIndex_r4/Slice  nbits=288  boolean_output=off  mode=Upper Bit Location + Width  bit1=-576  base1=MSB of Input  bit0=0  base0=LSB of Input  dbl_ovrd=off  block_type=slice
  slice3 : xbsIndex_r4/Slice  nbits=288  boolean_output=off  mode=Upper Bit Location + Width  bit1=-288  base1=MSB of Input  bit0=0  base0=LSB of Input  dbl_ovrd=off  block_type=slice
  slice4 : xbsIndex_r4/Slice  nbits=288  boolean_output=off  mode=Upper Bit Location + Width  bit1=0  base1=MSB of Input  bit0=0  base0=LSB of Input  dbl_ovrd=off  block_type=slice
  msb_out4 : Outport  VectorParamsAs1DForOutWhenUnconnected=off
  out3 : Outport  Port=2  VectorParamsAs1DForOutWhenUnconnected=off
  out2 : Outport  Port=3  VectorParamsAs1DForOutWhenUnconnected=off
  lsb_out1 : Outport  Port=4  VectorParamsAs1DForOutWhenUnconnected=off
  wires:
  bus_in/1 -> slice4/1, slice3/1, slice2/1, slice1/1
  slice4/1 -> reinterpret4/1
  reinterpret4/1 -> msb_out4/1
  slice3/1 -> reinterpret3/1
  reinterpret3/1 -> out3/1
  slice2/1 -> reinterpret2/1
  reinterpret2/1 -> out2/1
  slice1/1 -> reinterpret1/1
  reinterpret1/1 -> lsb_out1/1
